simulink model "SOC_2023b"  (R2023b)
settings: Solver=VariableStepAuto  StartTime=0.0  StopTime=10.0  RelTol=1e-3
undefined symbols (not defined in the file or in its folder): Initial_SOC
(part 2 of 187)

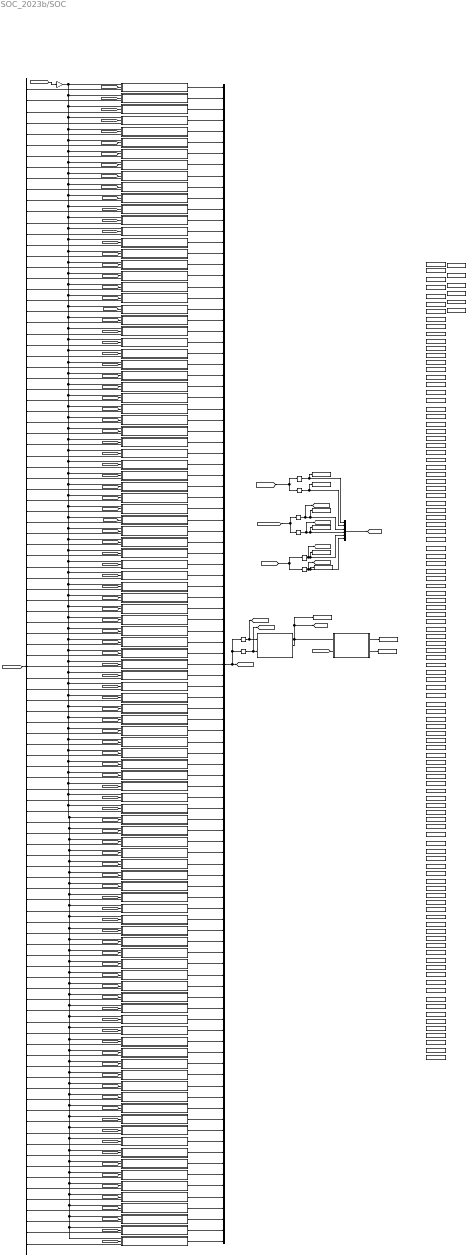
== system SOC_2023b/SOC ==
  note: 34 Cells in Parallel
  note: McMaster Automotive Resource Centre (MARC)
      | Electrical Engineer: Romulo Vieira, M.Sc and MBA
  note: State of Charge - Store Variables
  Bus Creator1 : BusCreator  Inputs=6
  Bus Creator2 : BusCreator  Inputs=105
  Bus Selector2 : BusSelector  OutputSignals=Cell Temperature 1,Cell Temperature 2,Cell Temperature 3,Cell Temperature 4,Cell Temperature 5,Cell Temperature 6,Cell Temperature 7,Cell Temperature 8,Cell Temperature 9,Cell Temperature 10,Cell Temperature 11,Cell Temperature 12,Cell Temperature 13,Cell Temperature 14,Cell Temperature 15,Cell Temperature 16,Cell Temperature 17,Cell Temperature 18,Cell Temperature 19,Cell Temperature 20,Cell Temperature 21,Cell Temperature 22,Cell Temperature 23,Cell Temperature 24,Cell Temperature 25,Cell Temperature 26,Cell Temperature 27,Cell Temperature 28,Cell Temperature 29,Cell Temperature 30,Cell Temperature 31,Cell Temperature 32,Cell Temperature 33,Cell Temperature 34,Cell Temperature 35,Cell Temperature 36,Cell Temperature 37,Cell Temperature 38,Cell Temperature 39,Cell Temperature 40,Cell Temperature 41,Cell Temperature 42,Cell Temperature 43,Cell Temperature 44,Cell Temperature 45,Cell Temperature 46,Cell Temperature 47,Cell Temperature 48,Cell Temperature 49,Cell Temperature 50,Cell Temperature 51,Cell Temperature 52,Cell Temperature 53,Cell Temperature 54,Cell Temperature 55,Cell Temperature 56,Cell Temperature 57,Cell Temperature 58,Cell Temperature 59,Cell Temperature 60,Cell Temperature 61,Cell Temperature 62,Cell Temperature 63,Cell Temperature 64,Cell Temperature 65,Cell Temperature 66,Cell Temperature 67,Cell Temperature 68,Cell Temperature 69,Cell Temperature 70,Cell Temperature 71,Cell Temperature 72,Cell Temperature 73,Cell Temperature 74,Cell Temperature 75,Cell Temperature 76,Cell Temperature 77,Cell Temperature 78,Cell Temperature 79,Cell Temperature 80,Cell Temperature 81,Cell Temperature 82,Cell Temperature 83,Cell Temperature 84,Cell Temperature 85,Cell Temperature 86,Cell Temperature 87,Cell Temperature 88,Cell Temperature 89,Cell Temperature 90,Cell Temperature 91,Cell Temperature 92,Cell Temperature 93,Cell Temperature 94,Cell Temperature 95,Cell Temperature 66,Cell Temperature 97,Cell Temperature 98,Cell Temperature 99,Cell Temperature 100,Cell Temperature 101,Cell Temperature 102,Cell Temperature 103,Cell Temperature 87,Cell Temperature 105
  Data Store Memory1 : DataStoreMemory  DataStoreName=HVBatSOC  ReadBeforeWriteMsg=warning  WriteAfterWriteMsg=warning  WriteAfterReadMsg=warning  ShowAdditionalParam=off  OutDataTypeStr=double
  Data Store Memory10 : DataStoreMemory  DataStoreName=HVBat_SOCcell014  ReadBeforeWriteMsg=warning  WriteAfterWriteMsg=warning  WriteAfterReadMsg=warning  ShowAdditionalParam=off  OutDataTypeStr=double
  Data Store Memory100 : DataStoreMemory  DataStoreName=HVBat_SOCcell097  ReadBeforeWriteMsg=warning  WriteAfterWriteMsg=warning  WriteAfterReadMsg=warning  ShowAdditionalParam=off  OutDataTypeStr=double
  Data Store Memory101 : DataStoreMemory  DataStoreName=HVBat_SOCcell098  ReadBeforeWriteMsg=warning  WriteAfterWriteMsg=warning  WriteAfterReadMsg=warning  ShowAdditionalParam=off  OutDataTypeStr=double
  Data Store Memory102 : DataStoreMemory  DataStoreName=HVBat_SOCcell099  ReadBeforeWriteMsg=warning  WriteAfterWriteMsg=warning  WriteAfterReadMsg=warning  ShowAdditionalParam=off  OutDataTypeStr=double
  Data Store Memory103 : DataStoreMemory  DataStoreName=HVBat_SOCcell100  ReadBeforeWriteMsg=warning  WriteAfterWriteMsg=warning  WriteAfterReadMsg=warning  ShowAdditionalParam=off  OutDataTypeStr=double
  Data Store Memory104 : DataStoreMemory  DataStoreName=HVBat_SOCcell101  ReadBeforeWriteMsg=warning  WriteAfterWriteMsg=warning  WriteAfterReadMsg=warning  ShowAdditionalParam=off  OutDataTypeStr=double
  Data Store Memory105 : DataStoreMemory  DataStoreName=HVBatTempMin  ReadBeforeWriteMsg=warning  WriteAfterWriteMsg=warning  WriteAfterReadMsg=warning  ShowAdditionalParam=off  OutDataTypeStr=double
  Data Store Memory106 : DataStoreMemory  DataStoreName=HVBatTempMax  ReadBeforeWriteMsg=warning  WriteAfterWriteMsg=warning  WriteAfterReadMsg=warning  ShowAdditionalParam=off  OutDataTypeStr=double
  Data Store Memory107 : DataStoreMemory  DataStoreName=HVBat_SOCcell089  ReadBeforeWriteMsg=warning  WriteAfterWriteMsg=warning  WriteAfterReadMsg=warning  ShowAdditionalParam=off  OutDataTypeStr=double
  Data Store Memory108 : DataStoreMemory  DataStoreName=HVBat_SOCcell090  ReadBeforeWriteMsg=warning  WriteAfterWriteMsg=warning  WriteAfterReadMsg=warning  ShowAdditionalParam=off  OutDataTypeStr=double
  Data Store Memory109 : DataStoreMemory  DataStoreName=HVBat_SOCcell091  ReadBeforeWriteMsg=warning  WriteAfterWriteMsg=warning  WriteAfterReadMsg=warning  ShowAdditionalParam=off  OutDataTypeStr=double
  Data Store Memory11 : DataStoreMemory  DataStoreName=HVBat_SOCcell028  ReadBeforeWriteMsg=warning  WriteAfterWriteMsg=warning  WriteAfterReadMsg=warning  ShowAdditionalParam=off  OutDataTypeStr=double
  Data Store Memory110 : DataStoreMemory  DataStoreName=HVBat_SOCcell092  ReadBeforeWriteMsg=warning  WriteAfterWriteMsg=warning  WriteAfterReadMsg=warning  ShowAdditionalParam=off  OutDataTypeStr=double
  Data Store Memory111 : DataStoreMemory  DataStoreName=HVBat_SOCcell093  ReadBeforeWriteMsg=warning  WriteAfterWriteMsg=warning  WriteAfterReadMsg=warning  ShowAdditionalParam=off  OutDataTypeStr=double
  Data Store Memory112 : DataStoreMemory  DataStoreName=HVBatVoltMax  ReadBeforeWriteMsg=warning  WriteAfterWriteMsg=warning  WriteAfterReadMsg=warning  ShowAdditionalParam=off  OutDataTypeStr=double
  Data Store Memory113 : DataStoreMemory  DataStoreName=HVBatVoltMin  ReadBeforeWriteMsg=warning  WriteAfterWriteMsg=warning  WriteAfterReadMsg=warning  ShowAdditionalParam=off  OutDataTypeStr=double
  Data Store Memory114 : DataStoreMemory  DataStoreName=HVBatSOCMin  ReadBeforeWriteMsg=warning  WriteAfterWriteMsg=warning  WriteAfterReadMsg=warning  ShowAdditionalParam=off  OutDataTypeStr=double
  Data Store Memory12 : DataStoreMemory  DataStoreName=HVBat_SOCcell022  ReadBeforeWriteMsg=warning  WriteAfterWriteMsg=warning  WriteAfterReadMsg=warning  ShowAdditionalParam=off  OutDataTypeStr=double
  Data Store Memory13 : DataStoreMemory  DataStoreName=HVBat_SOCcell023  ReadBeforeWriteMsg=warning  WriteAfterWriteMsg=warning  WriteAfterReadMsg=warning  ShowAdditionalParam=off  OutDataTypeStr=double
  Data Store Memory14 : DataStoreMemory  DataStoreName=HVBat_SOCcell016  ReadBeforeWriteMsg=warning  WriteAfterWriteMsg=warning  WriteAfterReadMsg=warning  ShowAdditionalParam=off  OutDataTypeStr=double
  Data Store Memory15 : DataStoreMemory  DataStoreName=HVBat_SOCcell024  ReadBeforeWriteMsg=warning  WriteAfterWriteMsg=warning  WriteAfterReadMsg=warning  ShowAdditionalParam=off  OutDataTypeStr=double
  Data Store Memory16 : DataStoreMemory  DataStoreName=HVBat_SOCcell025  ReadBeforeWriteMsg=warning  WriteAfterWriteMsg=warning  WriteAfterReadMsg=warning  ShowAdditionalParam=off  OutDataTypeStr=double
  Data Store Memory17 : DataStoreMemory  DataStoreName=HVBat_SOCcell015  ReadBeforeWriteMsg=warning  WriteAfterWriteMsg=warning  WriteAfterReadMsg=warning  ShowAdditionalParam=off  OutDataTypeStr=double
  Data Store Memory18 : DataStoreMemory  DataStoreName=HVBat_SOCcell017  ReadBeforeWriteMsg=warning  WriteAfterWriteMsg=warning  WriteAfterReadMsg=warning  ShowAdditionalParam=off  OutDataTypeStr=double
  Data Store Memory19 : DataStoreMemory  DataStoreName=HVBat_SOCcell018  ReadBeforeWriteMsg=warning  WriteAfterWriteMsg=warning  WriteAfterReadMsg=warning  ShowAdditionalParam=off  OutDataTypeStr=double
  Data Store Memory2 : DataStoreMemory  DataStoreName=HVBatFull_Amp_Hr_Capacity  ReadBeforeWriteMsg=warning  WriteAfterWriteMsg=warning  WriteAfterReadMsg=warning  ShowAdditionalParam=off  OutDataTypeStr=double
  Data Store Memory20 : DataStoreMemory  DataStoreName=HVBat_SOCcell019  ReadBeforeWriteMsg=warning  WriteAfterWriteMsg=warning  WriteAfterReadMsg=warning  ShowAdditionalParam=off  OutDataTypeStr=double
  Data Store Memory21 : DataStoreMemory  DataStoreName=HVBat_SOCcell020  ReadBeforeWriteMsg=warning  WriteAfterWriteMsg=warning  WriteAfterReadMsg=warning  ShowAdditionalParam=off  OutDataTypeStr=double
  Data Store Memory22 : DataStoreMemory  DataStoreName=HVBat_SOCcell021  ReadBeforeWriteMsg=warning  WriteAfterWriteMsg=warning  WriteAfterReadMsg=warning  ShowAdditionalParam=off  OutDataTypeStr=double
  Data Store Memory23 : DataStoreMemory  DataStoreName=HVBat_SOCcell026  ReadBeforeWriteMsg=warning  WriteAfterWriteMsg=warning  WriteAfterReadMsg=warning  ShowAdditionalParam=off  OutDataTypeStr=double
  Data Store Memory24 : DataStoreMemory  DataStoreName=HVBat_SOCcell027  ReadBeforeWriteMsg=warning  WriteAfterWriteMsg=warning  WriteAfterReadMsg=warning  ShowAdditionalParam=off  OutDataTypeStr=double
  Data Store Memory25 : DataStoreMemory  DataStoreName=HVBat_SOCcell034  ReadBeforeWriteMsg=warning  WriteAfterWriteMsg=warning  WriteAfterReadMsg=warning  ShowAdditionalParam=off  OutDataTypeStr=double
  Data Store Memory26 : DataStoreMemory  DataStoreName=HVBat_SOCcell048  ReadBeforeWriteMsg=warning  WriteAfterWriteMsg=warning  WriteAfterReadMsg=warning  ShowAdditionalParam=off  OutDataTypeStr=double
  Data Store Memory27 : DataStoreMemory  DataStoreName=HVBat_SOCcell042  ReadBeforeWriteMsg=warning  WriteAfterWriteMsg=warning  WriteAfterReadMsg=warning  ShowAdditionalParam=off  OutDataTypeStr=double
  Data Store Memory28 : DataStoreMemory  DataStoreName=HVBat_SOCcell043  ReadBeforeWriteMsg=warning  WriteAfterWriteMsg=warning  WriteAfterReadMsg=warning  ShowAdditionalParam=off  OutDataTypeStr=double
  Data Store Memory29 : DataStoreMemory  DataStoreName=HVBat_SOCcell036  ReadBeforeWriteMsg=warning  WriteAfterWriteMsg=warning  WriteAfterReadMsg=warning  ShowAdditionalParam=off  OutDataTypeStr=double
  Data Store Memory3 : DataStoreMemory  DataStoreName=HVBat_Energy  ReadBeforeWriteMsg=warning  WriteAfterWriteMsg=warning  WriteAfterReadMsg=warning  ShowAdditionalParam=off  OutDataTypeStr=double
  Data Store Memory30 : DataStoreMemory  DataStoreName=HVBat_SOCcell044  ReadBeforeWriteMsg=warning  WriteAfterWriteMsg=warning  WriteAfterReadMsg=warning  ShowAdditionalParam=off  OutDataTypeStr=double
  Data Store Memory31 : DataStoreMemory  DataStoreName=HVBat_SOCcell045  ReadBeforeWriteMsg=warning  WriteAfterWriteMsg=warning  WriteAfterReadMsg=warning  ShowAdditionalParam=off  OutDataTypeStr=double
  Data Store Memory32 : DataStoreMemory  DataStoreName=HVBat_SOCcell035  ReadBeforeWriteMsg=warning  WriteAfterWriteMsg=warning  WriteAfterReadMsg=warning  ShowAdditionalParam=off  OutDataTypeStr=double
  Data Store Memory33 : DataStoreMemory  DataStoreName=HVBat_SOCcell037  ReadBeforeWriteMsg=warning  WriteAfterWriteMsg=warning  WriteAfterReadMsg=warning  ShowAdditionalParam=off  OutDataTypeStr=double
  Data Store Memory34 : DataStoreMemory  DataStoreName=HVBat_SOCcell038  ReadBeforeWriteMsg=warning  WriteAfterWriteMsg=warning  WriteAfterReadMsg=warning  ShowAdditionalParam=off  OutDataTypeStr=double
  Data Store Memory35 : DataStoreMemory  DataStoreName=HVBat_SOCcell039  ReadBeforeWriteMsg=warning  WriteAfterWriteMsg=warning  WriteAfterReadMsg=warning  ShowAdditionalParam=off  OutDataTypeStr=double
  Data Store Memory36 : DataStoreMemory  DataStoreName=HVBat_SOCcell040  ReadBeforeWriteMsg=warning  WriteAfterWriteMsg=warning  WriteAfterReadMsg=warning  ShowAdditionalParam=off  OutDataTypeStr=double
  Data Store Memory37 : DataStoreMemory  DataStoreName=HVBat_SOCcell041  ReadBeforeWriteMsg=warning  WriteAfterWriteMsg=warning  WriteAfterReadMsg=warning  ShowAdditionalParam=off  OutDataTypeStr=double
  Data Store Memory38 : DataStoreMemory  DataStoreName=HVBat_SOCcell046  ReadBeforeWriteMsg=warning  WriteAfterWriteMsg=warning  WriteAfterReadMsg=warning  ShowAdditionalParam=off  OutDataTypeStr=double
  Data Store Memory39 : DataStoreMemory  DataStoreName=HVBat_SOCcell047  ReadBeforeWriteMsg=warning  WriteAfterWriteMsg=warning  WriteAfterReadMsg=warning  ShowAdditionalParam=off  OutDataTypeStr=double
  Data Store Memory4 : DataStoreMemory  DataStoreName=HVBat_SOCcell008  ReadBeforeWriteMsg=warning  WriteAfterWriteMsg=warning  WriteAfterReadMsg=warning  ShowAdditionalParam=off  OutDataTypeStr=double
  Data Store Memory40 : DataStoreMemory  DataStoreName=HVBat_SOCcell029  ReadBeforeWriteMsg=warning  WriteAfterWriteMsg=warning  WriteAfterReadMsg=warning  ShowAdditionalParam=off  OutDataTypeStr=double
  Data Store Memory41 : DataStoreMemory  DataStoreName=HVBat_SOCcell030  ReadBeforeWriteMsg=warning  WriteAfterWriteMsg=warning  WriteAfterReadMsg=warning  ShowAdditionalParam=off  OutDataTypeStr=double
  Data Store Memory42 : DataStoreMemory  DataStoreName=HVBat_SOCcell031  ReadBeforeWriteMsg=warning  WriteAfterWriteMsg=warning  WriteAfterReadMsg=warning  ShowAdditionalParam=off  OutDataTypeStr=double
  Data Store Memory43 : DataStoreMemory  DataStoreName=HVBat_SOCcell032  ReadBeforeWriteMsg=warning  WriteAfterWriteMsg=warning  WriteAfterReadMsg=warning  ShowAdditionalParam=off  OutDataTypeStr=double
  Data Store Memory44 : DataStoreMemory  DataStoreName=HVBat_SOCcell033  ReadBeforeWriteMsg=warning  WriteAfterWriteMsg=warning  WriteAfterReadMsg=warning  ShowAdditionalParam=off  OutDataTypeStr=double
  Data Store Memory45 : DataStoreMemory  DataStoreName=HVBat_SOCcell054  ReadBeforeWriteMsg=warning  WriteAfterWriteMsg=warning  WriteAfterReadMsg=warning  ShowAdditionalParam=off  OutDataTypeStr=double
  Data Store Memory46 : DataStoreMemory  DataStoreName=HVBat_SOCcell068  ReadBeforeWriteMsg=warning  WriteAfterWriteMsg=warning  WriteAfterReadMsg=warning  ShowAdditionalParam=off  OutDataTypeStr=double
  Data Store Memory47 : DataStoreMemory  DataStoreName=HVBat_SOCcell062  ReadBeforeWriteMsg=warning  WriteAfterWriteMsg=warning  WriteAfterReadMsg=warning  ShowAdditionalParam=off  OutDataTypeStr=double
  Data Store Memory48 : DataStoreMemory  DataStoreName=HVBat_SOCcell063  ReadBeforeWriteMsg=warning  WriteAfterWriteMsg=warning  WriteAfterReadMsg=warning  ShowAdditionalParam=off  OutDataTypeStr=double
  Data Store Memory49 : DataStoreMemory  DataStoreName=HVBat_SOCcell056  ReadBeforeWriteMsg=warning  WriteAfterWriteMsg=warning  WriteAfterReadMsg=warning  ShowAdditionalParam=off  OutDataTypeStr=double
  Data Store Memory5 : DataStoreMemory  DataStoreName=HVBat_SOCcell009  ReadBeforeWriteMsg=warning  WriteAfterWriteMsg=warning  WriteAfterReadMsg=warning  ShowAdditionalParam=off  OutDataTypeStr=double
  Data Store Memory50 : DataStoreMemory  DataStoreName=HVBat_SOCcell064  ReadBeforeWriteMsg=warning  WriteAfterWriteMsg=warning  WriteAfterReadMsg=warning  ShowAdditionalParam=off  OutDataTypeStr=double
  Data Store Memory51 : DataStoreMemory  DataStoreName=HVBat_SOCcell065  ReadBeforeWriteMsg=warning  WriteAfterWriteMsg=warning  WriteAfterReadMsg=warning  ShowAdditionalParam=off  OutDataTypeStr=double
  Data Store Memory52 : DataStoreMemory  DataStoreName=HVBat_SOCcell055  ReadBeforeWriteMsg=warning  WriteAfterWriteMsg=warning  WriteAfterReadMsg=warning  ShowAdditionalParam=off  OutDataTypeStr=double
  Data Store Memory53 : DataStoreMemory  DataStoreName=HVBat_SOCcell057  ReadBeforeWriteMsg=warning  WriteAfterWriteMsg=warning  WriteAfterReadMsg=warning  ShowAdditionalParam=off  OutDataTypeStr=double
  Data Store Memory54 : DataStoreMemory  DataStoreName=HVBat_SOCcell058  ReadBeforeWriteMsg=warning  WriteAfterWriteMsg=warning  WriteAfterReadMsg=warning  ShowAdditionalParam=off  OutDataTypeStr=double
  Data Store Memory55 : DataStoreMemory  DataStoreName=HVBat_SOCcell059  ReadBeforeWriteMsg=warning  WriteAfterWriteMsg=warning  WriteAfterReadMsg=warning  ShowAdditionalParam=off  OutDataTypeStr=double
  Data Store Memory56 : DataStoreMemory  DataStoreName=HVBat_SOCcell002  ReadBeforeWriteMsg=warning  WriteAfterWriteMsg=warning  WriteAfterReadMsg=warning  ShowAdditionalParam=off  OutDataTypeStr=double
  Data Store Memory57 : DataStoreMemory  DataStoreName=HVBat_SOCcell060  ReadBeforeWriteMsg=warning  WriteAfterWriteMsg=warning  WriteAfterReadMsg=warning  ShowAdditionalParam=off  OutDataTypeStr=double
  Data Store Memory58 : DataStoreMemory  DataStoreName=HVBat_SOCcell061  ReadBeforeWriteMsg=warning  WriteAfterWriteMsg=warning  WriteAfterReadMsg=warning  ShowAdditionalParam=off  OutDataTypeStr=double
  Data Store Memory59 : DataStoreMemory  DataStoreName=HVBat_SOCcell066  ReadBeforeWriteMsg=warning  WriteAfterWriteMsg=warning  WriteAfterReadMsg=warning  ShowAdditionalParam=off  OutDataTypeStr=double
  Data Store Memory6 : DataStoreMemory  DataStoreName=HVBat_SOCcell010  ReadBeforeWriteMsg=warning  WriteAfterWriteMsg=warning  WriteAfterReadMsg=warning  ShowAdditionalParam=off  OutDataTypeStr=double
  Data Store Memory60 : DataStoreMemory  DataStoreName=HVBat_SOCcell067  ReadBeforeWriteMsg=warning  WriteAfterWriteMsg=warning  WriteAfterReadMsg=warning  ShowAdditionalParam=off  OutDataTypeStr=double
  Data Store Memory61 : DataStoreMemory  DataStoreName=HVBat_SOCcell049  ReadBeforeWriteMsg=warning  WriteAfterWriteMsg=warning  WriteAfterReadMsg=warning  ShowAdditionalParam=off  OutDataTypeStr=double
  Data Store Memory62 : DataStoreMemory  DataStoreName=HVBat_SOCcell050  ReadBeforeWriteMsg=warning  WriteAfterWriteMsg=warning  WriteAfterReadMsg=warning  ShowAdditionalParam=off  OutDataTypeStr=double
  Data Store Memory63 : DataStoreMemory  DataStoreName=HVBat_SOCcell051  ReadBeforeWriteMsg=warning  WriteAfterWriteMsg=warning  WriteAfterReadMsg=warning  ShowAdditionalParam=off  OutDataTypeStr=double
  Data Store Memory64 : DataStoreMemory  DataStoreName=HVBat_SOCcell052  ReadBeforeWriteMsg=warning  WriteAfterWriteMsg=warning  WriteAfterReadMsg=warning  ShowAdditionalParam=off  OutDataTypeStr=double
  Data Store Memory65 : DataStoreMemory  DataStoreName=HVBat_SOCcell053  ReadBeforeWriteMsg=warning  WriteAfterWriteMsg=warning  WriteAfterReadMsg=warning  ShowAdditionalParam=off  OutDataTypeStr=double
  Data Store Memory66 : DataStoreMemory  DataStoreName=HVBat_SOCcell074  ReadBeforeWriteMsg=warning  WriteAfterWriteMsg=warning  WriteAfterReadMsg=warning  ShowAdditionalParam=off  OutDataTypeStr=double
  Data Store Memory67 : DataStoreMemory  DataStoreName=HVBat_SOCcell088  ReadBeforeWriteMsg=warning  WriteAfterWriteMsg=warning  WriteAfterReadMsg=warning  ShowAdditionalParam=off  OutDataTypeStr=double
  Data Store Memory68 : DataStoreMemory  DataStoreName=HVBat_SOCcell082  ReadBeforeWriteMsg=warning  WriteAfterWriteMsg=warning  WriteAfterReadMsg=warning  ShowAdditionalParam=off  OutDataTypeStr=double
  Data Store Memory69 : DataStoreMemory  DataStoreName=HVBat_SOCcell083  ReadBeforeWriteMsg=warning  WriteAfterWriteMsg=warning  WriteAfterReadMsg=warning  ShowAdditionalParam=off  OutDataTypeStr=double
  Data Store Memory7 : DataStoreMemory  DataStoreName=HVBat_SOCcell011  ReadBeforeWriteMsg=warning  WriteAfterWriteMsg=warning  WriteAfterReadMsg=warning  ShowAdditionalParam=off  OutDataTypeStr=double
  Data Store Memory70 : DataStoreMemory  DataStoreName=HVBat_SOCcell076  ReadBeforeWriteMsg=warning  WriteAfterWriteMsg=warning  WriteAfterReadMsg=warning  ShowAdditionalParam=off  OutDataTypeStr=double
  Data Store Memory71 : DataStoreMemory  DataStoreName=HVBat_SOCcell084  ReadBeforeWriteMsg=warning  WriteAfterWriteMsg=warning  WriteAfterReadMsg=warning  ShowAdditionalParam=off  OutDataTypeStr=double
  Data Store Memory72 : DataStoreMemory  DataStoreName=HVBat_SOCcell085  ReadBeforeWriteMsg=warning  WriteAfterWriteMsg=warning  WriteAfterReadMsg=warning  ShowAdditionalParam=off  OutDataTypeStr=double
  Data Store Memory73 : DataStoreMemory  DataStoreName=HVBat_SOCcell001  ReadBeforeWriteMsg=warning  WriteAfterWriteMsg=warning  WriteAfterReadMsg=warning  ShowAdditionalParam=off  OutDataTypeStr=double
  Data Store Memory74 : DataStoreMemory  DataStoreName=HVBat_SOCcell003  ReadBeforeWriteMsg=warning  WriteAfterWriteMsg=warning  WriteAfterReadMsg=warning  ShowAdditionalParam=off  OutDataTypeStr=double
  Data Store Memory75 : DataStoreMemory  DataStoreName=HVBat_SOCcell004  ReadBeforeWriteMsg=warning  WriteAfterWriteMsg=warning  WriteAfterReadMsg=warning  ShowAdditionalParam=off  OutDataTypeStr=double
  Data Store Memory76 : DataStoreMemory  DataStoreName=HVBat_SOCcell005  ReadBeforeWriteMsg=warning  WriteAfterWriteMsg=warning  WriteAfterReadMsg=warning  ShowAdditionalParam=off  OutDataTypeStr=double
  Data Store Memory77 : DataStoreMemory  DataStoreName=HVBat_SOCcell006  ReadBeforeWriteMsg=warning  WriteAfterWriteMsg=warning  WriteAfterReadMsg=warning  ShowAdditionalParam=off  OutDataTypeStr=double
  Data Store Memory78 : DataStoreMemory  DataStoreName=HVBat_SOCcell007  ReadBeforeWriteMsg=warning  WriteAfterWriteMsg=warning  WriteAfterReadMsg=warning  ShowAdditionalParam=off  OutDataTypeStr=double
  Data Store Memory79 : DataStoreMemory  DataStoreName=HVBat_SOCcell075  ReadBeforeWriteMsg=warning  WriteAfterWriteMsg=warning  WriteAfterReadMsg=warning  ShowAdditionalParam=off  OutDataTypeStr=double
  Data Store Memory8 : DataStoreMemory  DataStoreName=HVBat_SOCcell012  ReadBeforeWriteMsg=warning  WriteAfterWriteMsg=warning  WriteAfterReadMsg=warning  ShowAdditionalParam=off  OutDataTypeStr=double
  Data Store Memory80 : DataStoreMemory  DataStoreName=HVBat_SOCcell077  ReadBeforeWriteMsg=warning  WriteAfterWriteMsg=warning  WriteAfterReadMsg=warning  ShowAdditionalParam=off  OutDataTypeStr=double
  Data Store Memory81 : DataStoreMemory  DataStoreName=HVBat_SOCcell078  ReadBeforeWriteMsg=warning  WriteAfterWriteMsg=warning  WriteAfterReadMsg=warning  ShowAdditionalParam=off  OutDataTypeStr=double
  Data Store Memory82 : DataStoreMemory  DataStoreName=HVBat_SOCcell079  ReadBeforeWriteMsg=warning  WriteAfterWriteMsg=warning  WriteAfterReadMsg=warning  ShowAdditionalParam=off  OutDataTypeStr=double
  Data Store Memory83 : DataStoreMemory  DataStoreName=HVBat_SOCcell080  ReadBeforeWriteMsg=warning  WriteAfterWriteMsg=warning  WriteAfterReadMsg=warning  ShowAdditionalParam=off  OutDataTypeStr=double
  Data Store Memory84 : DataStoreMemory  DataStoreName=HVBat_SOCcell081  ReadBeforeWriteMsg=warning  WriteAfterWriteMsg=warning  WriteAfterReadMsg=warning  ShowAdditionalParam=off  OutDataTypeStr=double
  Data Store Memory85 : DataStoreMemory  DataStoreName=HVBat_SOCcell086  ReadBeforeWriteMsg=warning  WriteAfterWriteMsg=warning  WriteAfterReadMsg=warning  ShowAdditionalParam=off  OutDataTypeStr=double
  Data Store Memory86 : DataStoreMemory  DataStoreName=HVBat_SOCcell087  ReadBeforeWriteMsg=warning  WriteAfterWriteMsg=warning  WriteAfterReadMsg=warning  ShowAdditionalParam=off  OutDataTypeStr=double
  Data Store Memory87 : DataStoreMemory  DataStoreName=HVBat_SOCcell069  ReadBeforeWriteMsg=warning  WriteAfterWriteMsg=warning  WriteAfterReadMsg=warning  ShowAdditionalParam=off  OutDataTypeStr=double
  Data Store Memory88 : DataStoreMemory  DataStoreName=HVBat_SOCcell070  ReadBeforeWriteMsg=warning  WriteAfterWriteMsg=warning  WriteAfterReadMsg=warning  ShowAdditionalParam=off  OutDataTypeStr=double
  Data Store Memory89 : DataStoreMemory  DataStoreName=HVBat_SOCcell071  ReadBeforeWriteMsg=warning  WriteAfterWriteMsg=warning  WriteAfterReadMsg=warning  ShowAdditionalParam=off  OutDataTypeStr=double
  Data Store Memory9 : DataStoreMemory  DataStoreName=HVBat_SOCcell013  ReadBeforeWriteMsg=warning  WriteAfterWriteMsg=warning  WriteAfterReadMsg=warning  ShowAdditionalParam=off  OutDataTypeStr=double
  Data Store Memory90 : DataStoreMemory  DataStoreName=HVBat_SOCcell072  ReadBeforeWriteMsg=warning  WriteAfterWriteMsg=warning  WriteAfterReadMsg=warning  ShowAdditionalParam=off  OutDataTypeStr=double
  Data Store Memory91 : DataStoreMemory  DataStoreName=HVBat_SOCcell073  ReadBeforeWriteMsg=warning  WriteAfterWriteMsg=warning  WriteAfterReadMsg=warning  ShowAdditionalParam=off  OutDataTypeStr=double
  Data Store Memory92 : DataStoreMemory  DataStoreName=HVBat_SOCcell094  ReadBeforeWriteMsg=warning  WriteAfterWriteMsg=warning  WriteAfterReadMsg=warning  ShowAdditionalParam=off  OutDataTypeStr=double
  Data Store Memory93 : DataStoreMemory  DataStoreName=HVBatSOCMax  ReadBeforeWriteMsg=warning  WriteAfterWriteMsg=warning  WriteAfterReadMsg=warning  ShowAdditionalParam=off  OutDataTypeStr=double
  Data Store Memory94 : DataStoreMemory  DataStoreName=HVBat_SOCcell102  ReadBeforeWriteMsg=warning  WriteAfterWriteMsg=warning  WriteAfterReadMsg=warning  ShowAdditionalParam=off  OutDataTypeStr=double
  Data Store Memory95 : DataStoreMemory  DataStoreName=HVBat_SOCcell103  ReadBeforeWriteMsg=warning  WriteAfterWriteMsg=warning  WriteAfterReadMsg=warning  ShowAdditionalParam=off  OutDataTypeStr=double
  Data Store Memory96 : DataStoreMemory  DataStoreName=HVBat_SOCcell096  ReadBeforeWriteMsg=warning  WriteAfterWriteMsg=warning  WriteAfterReadMsg=warning  ShowAdditionalParam=off  OutDataTypeStr=double
  Data Store Memory97 : DataStoreMemory  DataStoreName=HVBat_SOCcell104  ReadBeforeWriteMsg=warning  WriteAfterWriteMsg=warning  WriteAfterReadMsg=warning  ShowAdditionalParam=off  OutDataTypeStr=double
  Data Store Memory98 : DataStoreMemory  DataStoreName=HVBat_SOCcell105  ReadBeforeWriteMsg=warning  WriteAfterWriteMsg=warning  WriteAfterReadMsg=warning  ShowAdditionalParam=off  OutDataTypeStr=double
  Data Store Memory99 : DataStoreMemory  DataStoreName=HVBat_SOCcell095  ReadBeforeWriteMsg=warning  WriteAfterWriteMsg=warning  WriteAfterReadMsg=warning  ShowAdditionalParam=off  OutDataTypeStr=double
  Data Store Write1 : DataStoreWrite  DataStoreName=HVBatSOC  IndexOptions=Index vector (dialog)  Indices=1  OutputSizes=1
  Data Store Write10 : DataStoreWrite  DataStoreName=HVBatSOCMax  IndexOptions=Index vector (dialog)  Indices=1  OutputSizes=1
  Data Store Write11 : DataStoreWrite  DataStoreName=HVBatTempMin  IndexOptions=Index vector (dialog)  Indices=1  OutputSizes=1
  Data Store Write12 : DataStoreWrite  DataStoreName=HVBatTempMax  IndexOptions=Index vector (dialog)  Indices=1  OutputSizes=1
  Data Store Write13 : DataStoreWrite  DataStoreName=HVBatVoltMin  IndexOptions=Index vector (dialog)  Indices=1  OutputSizes=1
  Data Store Write14 : DataStoreWrite  DataStoreName=HVBatVoltMax  IndexOptions=Index vector (dialog)  Indices=1  OutputSizes=1
  Data Store Write2 : DataStoreWrite  DataStoreName=HVBatFull_Amp_Hr_Capacity  IndexOptions=Index vector (dialog)  Indices=1  OutputSizes=1
  Data Store Write3 : DataStoreWrite  DataStoreName=HVBat_Energy  IndexOptions=Index vector (dialog)  Indices=1  OutputSizes=1
  Data Store Write4 : DataStoreWrite  DataStoreName=HVBatSOCMin  IndexOptions=Index vector (dialog)  Indices=1  OutputSizes=1
  Gain1 : Gain  Gain=1/34
  MATLAB Function : MATLAB Function  TreatAsAtomicUnit=on
  MATLAB Function1 : MATLAB Function  TreatAsAtomicUnit=on
  Max{1..3,7} (4 alike) : MinMax
  Max{4..6,8} (4 alike) : MinMax  Function=max
  Multi-Temperature SOC EKF1 : Subsystem  TreatAsAtomicUnit=on  SystemSampleTime=1
  Multi-Temperature SOC EKF10 : Subsystem  TreatAsAtomicUnit=on  SystemSampleTime=1
  Multi-Temperature SOC EKF100 : Subsystem  TreatAsAtomicUnit=on  SystemSampleTime=1
  Multi-Temperature SOC EKF101 : Subsystem  TreatAsAtomicUnit=on  SystemSampleTime=1
  Multi-Temperature SOC EKF102 : Subsystem  TreatAsAtomicUnit=on  SystemSampleTime=1
  Multi-Temperature SOC EKF103 : Subsystem  TreatAsAtomicUnit=on  SystemSampleTime=1
  Multi-Temperature SOC EKF104 : Subsystem  TreatAsAtomicUnit=on  SystemSampleTime=1
  Multi-Temperature SOC EKF105 : Subsystem  TreatAsAtomicUnit=on  SystemSampleTime=1
  Multi-Temperature SOC EKF11 : Subsystem  TreatAsAtomicUnit=on  SystemSampleTime=1
  Multi-Temperature SOC EKF12 : Subsystem  TreatAsAtomicUnit=on  SystemSampleTime=1
  Multi-Temperature SOC EKF13 : Subsystem  TreatAsAtomicUnit=on  SystemSampleTime=1
  Multi-Temperature SOC EKF14 : Subsystem  TreatAsAtomicUnit=on  SystemSampleTime=1
  Multi-Temperature SOC EKF15 : Subsystem  TreatAsAtomicUnit=on  SystemSampleTime=1
  Multi-Temperature SOC EKF16 : Subsystem  TreatAsAtomicUnit=on  SystemSampleTime=1
  Multi-Temperature SOC EKF17 : Subsystem  TreatAsAtomicUnit=on  SystemSampleTime=1
  Multi-Temperature SOC EKF18 : Subsystem  TreatAsAtomicUnit=on  SystemSampleTime=1
  Multi-Temperature SOC EKF19 : Subsystem  TreatAsAtomicUnit=on  SystemSampleTime=1
  Multi-Temperature SOC EKF2 : Subsystem  TreatAsAtomicUnit=on  SystemSampleTime=1
  Multi-Temperature SOC EKF20 : Subsystem  TreatAsAtomicUnit=on  SystemSampleTime=1
  Multi-Temperature SOC EKF21 : Subsystem  TreatAsAtomicUnit=on  SystemSampleTime=1
  Multi-Temperature SOC EKF22 : Subsystem  TreatAsAtomicUnit=on  SystemSampleTime=1
  Multi-Temperature SOC EKF23 : Subsystem  TreatAsAtomicUnit=on  SystemSampleTime=1
  Multi-Temperature SOC EKF24 : Subsystem  TreatAsAtomicUnit=on  SystemSampleTime=1
  Multi-Temperature SOC EKF25 : Subsystem  TreatAsAtomicUnit=on  SystemSampleTime=1
  Multi-Temperature SOC EKF26 : Subsystem  TreatAsAtomicUnit=on  SystemSampleTime=1
  Multi-Temperature SOC EKF27 : Subsystem  TreatAsAtomicUnit=on  SystemSampleTime=1
  Multi-Temperature SOC EKF28 : Subsystem  TreatAsAtomicUnit=on  SystemSampleTime=1
  Multi-Temperature SOC EKF29 : Subsystem  TreatAsAtomicUnit=on  SystemSampleTime=1
  Multi-Temperature SOC EKF3 : Subsystem  TreatAsAtomicUnit=on  SystemSampleTime=1
  Multi-Temperature SOC EKF30 : Subsystem  TreatAsAtomicUnit=on  SystemSampleTime=1
  Multi-Temperature SOC EKF31 : Subsystem  TreatAsAtomicUnit=on  SystemSampleTime=1
  Multi-Temperature SOC EKF32 : Subsystem  TreatAsAtomicUnit=on  SystemSampleTime=1
  Multi-Temperature SOC EKF33 : Subsystem  TreatAsAtomicUnit=on  SystemSampleTime=1
  Multi-Temperature SOC EKF34 : Subsystem  TreatAsAtomicUnit=on  SystemSampleTime=1
  Multi-Temperature SOC EKF35 : Subsystem  TreatAsAtomicUnit=on  SystemSampleTime=1
  Multi-Temperature SOC EKF36 : Subsystem  TreatAsAtomicUnit=on  SystemSampleTime=1
  Multi-Temperature SOC EKF37 : Subsystem  TreatAsAtomicUnit=on  SystemSampleTime=1
  Multi-Temperature SOC EKF38 : Subsystem  TreatAsAtomicUnit=on  SystemSampleTime=1
  Multi-Temperature SOC EKF39 : Subsystem  TreatAsAtomicUnit=on  SystemSampleTime=1
  Multi-Temperature SOC EKF4 : Subsystem  TreatAsAtomicUnit=on  SystemSampleTime=1
  Multi-Temperature SOC EKF40 : Subsystem  TreatAsAtomicUnit=on  SystemSampleTime=1
  Multi-Temperature SOC EKF41 : Subsystem  TreatAsAtomicUnit=on  SystemSampleTime=1
  Multi-Temperature SOC EKF42 : Subsystem  TreatAsAtomicUnit=on  SystemSampleTime=1
  Multi-Temperature SOC EKF43 : Subsystem  TreatAsAtomicUnit=on  SystemSampleTime=1
  Multi-Temperature SOC EKF44 : Subsystem  TreatAsAtomicUnit=on  SystemSampleTime=1
  Multi-Temperature SOC EKF45 : Subsystem  TreatAsAtomicUnit=on  SystemSampleTime=1
  Multi-Temperature SOC EKF46 : Subsystem  TreatAsAtomicUnit=on  SystemSampleTime=1
  Multi-Temperature SOC EKF47 : Subsystem  TreatAsAtomicUnit=on  SystemSampleTime=1
  Multi-Temperature SOC EKF48 : Subsystem  TreatAsAtomicUnit=on  SystemSampleTime=1
  Multi-Temperature SOC EKF49 : Subsystem  TreatAsAtomicUnit=on  SystemSampleTime=1
  Multi-Temperature SOC EKF5 : Subsystem  TreatAsAtomicUnit=on  SystemSampleTime=1
  Multi-Temperature SOC EKF50 : Subsystem  TreatAsAtomicUnit=on  SystemSampleTime=1
  Multi-Temperature SOC EKF51 : Subsystem  TreatAsAtomicUnit=on  SystemSampleTime=1
  Multi-Temperature SOC EKF52 : Subsystem  TreatAsAtomicUnit=on  SystemSampleTime=1
  Multi-Temperature SOC EKF53 : Subsystem  TreatAsAtomicUnit=on  SystemSampleTime=1
  Multi-Temperature SOC EKF54 : Subsystem  TreatAsAtomicUnit=on  SystemSampleTime=1
  Multi-Temperature SOC EKF55 : Subsystem  TreatAsAtomicUnit=on  SystemSampleTime=1
  Multi-Temperature SOC EKF56 : Subsystem  TreatAsAtomicUnit=on  SystemSampleTime=1
  Multi-Temperature SOC EKF57 : Subsystem  TreatAsAtomicUnit=on  SystemSampleTime=1
  Multi-Temperature SOC EKF58 : Subsystem  TreatAsAtomicUnit=on  SystemSampleTime=1
  Multi-Temperature SOC EKF59 : Subsystem  TreatAsAtomicUnit=on  SystemSampleTime=1
  Multi-Temperature SOC EKF6 : Subsystem  TreatAsAtomicUnit=on  SystemSampleTime=1
  Multi-Temperature SOC EKF60 : Subsystem  TreatAsAtomicUnit=on  SystemSampleTime=1
  Multi-Temperature SOC EKF61 : Subsystem  TreatAsAtomicUnit=on  SystemSampleTime=1
  Multi-Temperature SOC EKF62 : Subsystem  TreatAsAtomicUnit=on  SystemSampleTime=1
  Multi-Temperature SOC EKF63 : Subsystem  TreatAsAtomicUnit=on  SystemSampleTime=1
  Multi-Temperature SOC EKF64 : Subsystem  TreatAsAtomicUnit=on  SystemSampleTime=1
  Multi-Temperature SOC EKF65 : Subsystem  TreatAsAtomicUnit=on  SystemSampleTime=1
  Multi-Temperature SOC EKF66 : Subsystem  TreatAsAtomicUnit=on  SystemSampleTime=1
  Multi-Temperature SOC EKF67 : Subsystem  TreatAsAtomicUnit=on  SystemSampleTime=1
  Multi-Temperature SOC EKF68 : Subsystem  TreatAsAtomicUnit=on  SystemSampleTime=1